simulink model "hyd_hyb_2_opt_cont_test_new_1_col_cd_fin_2"  (Simulink 7.7) (continued)
== system hyd_hyb_2_opt_cont_test_new_1_col_cd_fin_2/controller2/optimized controller/SOC upper 0.5/SOC upper 0.6/SOC upper 0.7/SOC under 0.8/net_loc_rod_7_8/Layer 2/LW{2,1} == (continued)
      mask Display: e = 0.06;c1 = [120 150 230]/255;c2 = ([120 150 230]+0)/383;c3 = ([120 150 230]+128)/383;patch([0 1 1 0],[0 0 1 1],c1);patch([0 1 1 1-e 1-e 0+e],[0 0 1 1-e 0+e 0+e],c2);patch([0 0 1 1-e 0+e 0+e],[0 1 1 1-e 1-e 0+e],c3);plot([0 1 1 0 0],[0 0 1 1 0]);disp('weights');
  "IW{2,1}(39,:)'" : Constant  Value=[-0.4835323749616523;0.31040278631905849;0.10047082836331186;-0.44502359853670193;0.39361966468221593;-0.34187227847267937;-0.18385257985250303;0.063961557191133347;-0.13311223909844291;-0.10037287921349862;-0.022734847026702414;0.32795556493781491;-0.20184661004759019;0.31043902538334567;0.48581448623302109;0.2923177940710513;-0.0082319895963805199;-0.23047279705256349;0.079154749884875836;-0.35425935283586912;-0.33585786795363404;0.55922628182483347;0.024927542637002982;-0.5369677098909581;-0.0095303478724389854;-0.21849709157034933;-0.21380711845089198;0.18488659257561837;0.37658112690975565;-0.098544098121421342]
      mask Display: e = 0.06;c1 = [120 150 230]/255;c2 = ([120 150 230]+0)/383;c3 = ([120 150 230]+128)/383;patch([0 1 1 0],[0 0 1 1],c1);patch([0 1 1 1-e 1-e 0+e],[0 0 1 1-e 0+e 0+e],c2);patch([0 0 1 1-e 0+e 0+e],[0 1 1 1-e 1-e 0+e],c3);plot([0 1 1 0 0],[0 0 1 1 0]);disp('weights');
  "IW{2,1}(4,:)'" : Constant  Value=[-0.084456356670670585;0.39332853492416614;-0.14093791023297814;-0.24699434973438217;0.037218999998135292;0.057290859811489341;-0.078827547784478064;0.49932008441699871;0.16387379971577443;-0.040748085545927626;-0.009324468468041516;-0.19160814023664818;-0.32053131109424393;-0.11908011228852317;-0.041753985083747211;0.26962081886530981;0.46929550230303646;-0.4728704361695264;0.068000938155538287;0.52995252288102757;-0.27876691608214754;0.34925409397203971;-0.023685241942146756;0.1027174484119477;-0.32882096042564896;0.5005590567264776;0.28540974607274233;-0.50723706812721148;0.21128315049192911;0.36676444229034771]
      mask Display: e = 0.06;c1 = [120 150 230]/255;c2 = ([120 150 230]+0)/383;c3 = ([120 150 230]+128)/383;patch([0 1 1 0],[0 0 1 1],c1);patch([0 1 1 1-e 1-e 0+e],[0 0 1 1-e 0+e 0+e],c2);patch([0 0 1 1-e 0+e 0+e],[0 1 1 1-e 1-e 0+e],c3);plot([0 1 1 0 0],[0 0 1 1 0]);disp('weights');
  "IW{2,1}(40,:)'" : Constant  Value=[0.11138375053922149;0.10725746152537521;-0.40441884661729288;-0.37853098423894987;-0.08157669713924344;-0.15157684402681071;0.028628353347344079;-0.43210093459350013;-0.44846964252056087;0.11118148102122939;0.1242481553642565;0.16879590214484513;0.41077554701764851;0.14755675464456003;0.21144023645213661;-0.20514570550553057;-0.44588331488494204;0.40541459720775785;-0.092804745325358151;-0.15342207436425032;0.097208902147965043;0.34979320899396732;0.33451829346632145;-0.16901843840161238;-0.39427988026929328;-0.36030296316647398;0.38700613792454092;-0.31771214900978895;-0.45420022589045494;-0.26445082825380611]
      mask Display: e = 0.06;c1 = [120 150 230]/255;c2 = ([120 150 230]+0)/383;c3 = ([120 150 230]+128)/383;patch([0 1 1 0],[0 0 1 1],c1);patch([0 1 1 1-e 1-e 0+e],[0 0 1 1-e 0+e 0+e],c2);patch([0 0 1 1-e 0+e 0+e],[0 1 1 1-e 1-e 0+e],c3);plot([0 1 1 0 0],[0 0 1 1 0]);disp('weights');
  "IW{2,1}(41,:)'" : Constant  Value=[-0.35630502406955966;-0.36974493824414606;0.15417390376028811;-0.38411362717766467;-0.35707706076629075;0.34165780337971213;0.14516683224012641;-0.21814421311647195;0.0032027169911279991;0.41624788464706797;-0.3397264421764124;-0.34898378374770811;-0.38215900969063754;-0.11404218518317454;-0.0050269406475612136;-0.098560432098857342;0.19486175046204038;0.32148116785508213;-0.38184911577693403;0.18293667936430713;-0.32949787704077893;0.35202275708123654;-0.36473945033324517;0.30077151631589694;0.2861707258441703;-0.080564081375307309;0.42006888197764058;0.11125025887557062;-0.24233566024583689;0.36772368597905775]
      mask Display: e = 0.06;c1 = [120 150 230]/255;c2 = ([120 150 230]+0)/383;c3 = ([120 150 230]+128)/383;patch([0 1 1 0],[0 0 1 1],c1);patch([0 1 1 1-e 1-e 0+e],[0 0 1 1-e 0+e 0+e],c2);patch([0 0 1 1-e 0+e 0+e],[0 1 1 1-e 1-e 0+e],c3);plot([0 1 1 0 0],[0 0 1 1 0]);disp('weights');
  "IW{2,1}(42,:)'" : Constant  Value=[0.15706169119829308;-0.34970464264246925;-0.091467553719374178;0.089096796137939638;0.37726052407804506;-0.45569306047604091;-0.40770262293161275;-0.23251625005436713;-0.25419916471996584;0.061556156981641227;0.38417584029606072;-0.20488679933615037;-0.11108397344155337;0.46208521453680423;-0.16681301652254793;0.009716834658453485;-0.31429738943502228;0.42058563389721992;0.39625395225088711;-0.32712238021736739;0.084481526805292709;-0.29846080219001225;0.07194158791340341;-0.47843218886833982;0.27604781968334097;0.087851162331685273;-0.42601946747014108;-0.17080271868571589;0.209081222714597;-0.25655431363119224]
      mask Display: e = 0.06;c1 = [120 150 230]/255;c2 = ([120 150 230]+0)/383;c3 = ([120 150 230]+128)/383;patch([0 1 1 0],[0 0 1 1],c1);patch([0 1 1 1-e 1-e 0+e],[0 0 1 1-e 0+e 0+e],c2);patch([0 0 1 1-e 0+e 0+e],[0 1 1 1-e 1-e 0+e],c3);plot([0 1 1 0 0],[0 0 1 1 0]);disp('weights');
  "IW{2,1}(43,:)'" : Constant  Value=[-0.31757640025894474;0.056653323308336612;-0.32544780806317231;0.3449113935863426;0.22500001165720365;0.066553259696957981;0.21248106162757274;0.096345459788785906;-0.34025605178291202;-0.44926824011835953;-0.33703884954801289;-0.39135850312812459;0.084811180139392969;0.29004102009119448;-0.066177614890907052;0.2824143243737593;-0.41299189747303039;0.23959623598881055;-0.36516527294692269;-0.029753147329238117;0.03851459755258807;-0.16463600865424638;0.2976532762799749;0.036542029343999097;0.44292641794603299;-0.31092484757236044;-0.14713729631980613;0.41061645318910794;-0.45692360248280139;0.38551952010652746]
      mask Display: e = 0.06;c1 = [120 150 230]/255;c2 = ([120 150 230]+0)/383;c3 = ([120 150 230]+128)/383;patch([0 1 1 0],[0 0 1 1],c1);patch([0 1 1 1-e 1-e 0+e],[0 0 1 1-e 0+e 0+e],c2);patch([0 0 1 1-e 0+e 0+e],[0 1 1 1-e 1-e 0+e],c3);plot([0 1 1 0 0],[0 0 1 1 0]);disp('weights');
  "IW{2,1}(44,:)'" : Constant  Value=[0.27056791623358634;0.08799096571828896;0.40404501597019188;0.22557856196010281;-0.24810705411037701;-0.46898974798625548;-0.48246857128286258;-0.018971563790798295;-0.14180175558241984;-0.11819502415132724;-0.26906558792700436;0.033084771748252563;0.49345840818175823;-0.38563447733884082;-0.24841433722989337;-0.20280384747095245;-0.35483187434085484;0.44982892766393817;0.14130516262448167;0.42433474138815308;-0.067420293627416056;-0.22114163016512606;-0.21059456031308763;-0.1947806306183705;0.082815646444673055;-0.25470852276293143;0.2875592759501811;0.39255425179318121;0.10175831647895807;-0.46482128911740023]
      mask Display: e = 0.06;c1 = [120 150 230]/255;c2 = ([120 150 230]+0)/383;c3 = ([120 150 230]+128)/383;patch([0 1 1 0],[0 0 1 1],c1);patch([0 1 1 1-e 1-e 0+e],[0 0 1 1-e 0+e 0+e],c2);patch([0 0 1 1-e 0+e 0+e],[0 1 1 1-e 1-e 0+e],c3);plot([0 1 1 0 0],[0 0 1 1 0]);disp('weights');
  "IW{2,1}(45,:)'" : Constant  Value=[-0.24278031243361256;0.16460435432975626;0.33695098142409952;-0.38763210309265494;0.11318182663408502;-0.24323292343715391;-0.35412139680927979;-0.48202397097123423;0.34298856029500091;0.45098885155589863;0.29640677932190812;0.12442504925656733;0.22785636922478969;-0.27471140977544156;-0.2568326759436787;-0.33040434674789931;0.40058542584282214;0.28838343142141509;-0.067936896110189549;-0.28159510671003796;0.078580146864540748;0.51250916638014188;0.32989365797735987;-0.26779269746604206;-0.255578957532062;-0.29727334764933683;0.13933585004022109;0.34436541155109002;-0.26846203565570803;-0.32946845076932563]
      mask Display: e = 0.06;c1 = [120 150 230]/255;c2 = ([120 150 230]+0)/383;c3 = ([120 150 230]+128)/383;patch([0 1 1 0],[0 0 1 1],c1);patch([0 1 1 1-e 1-e 0+e],[0 0 1 1-e 0+e 0+e],c2);patch([0 0 1 1-e 0+e 0+e],[0 1 1 1-e 1-e 0+e],c3);plot([0 1 1 0 0],[0 0 1 1 0]);disp('weights');
  "IW{2,1}(46,:)'" : Constant  Value=[0.47751372779563583;-0.004631941129258247;-0.26746797355239715;0.17883186503629808;-0.10548613834212665;0.13687902939422278;-0.40631954704837736;0.17093579645948126;0.095311678944684244;0.034110033251800002;0.3224740096213401;-0.15952180986137474;-0.31784651157245414;-0.45320379549388551;0.33045323818398969;0.22019799015671856;-0.35034858148840908;0.45625883071377721;-0.31719260036143726;-0.50139725511272237;0.1362457531355615;0.22618930416171001;-0.32470450433606052;-0.10633510402694961;-0.31408662865790887;-0.42624423653419202;0.15375441583054122;-0.12959182064022393;0.42217380252437764;-0.25530669324959132]
      mask Display: e = 0.06;c1 = [120 150 230]/255;c2 = ([120 150 230]+0)/383;c3 = ([120 150 230]+128)/383;patch([0 1 1 0],[0 0 1 1],c1);patch([0 1 1 1-e 1-e 0+e],[0 0 1 1-e 0+e 0+e],c2);patch([0 0 1 1-e 0+e 0+e],[0 1 1 1-e 1-e 0+e],c3);plot([0 1 1 0 0],[0 0 1 1 0]);disp('weights');
  "IW{2,1}(47,:)'" : Constant  Value=[-0.33058990973758129;-0.070122866651558854;-0.18964704315156067;0.15987361685157389;0.23026949273690664;0.44625637355610298;0.021772781753390227;-0.053731232580210356;0.08313635696579319;-0.35236494354447639;0.42953438289875867;0.075855817877353904;-0.39252730569594219;0.2648014400238784;0.33156686386675471;0.0039727953447689611;0.34232437544328292;0.42230368814878627;0.38625146824659068;0.37475339723843737;0.39234153591899412;-0.29576359594338791;0.044467362563895552;-0.18506463939778761;0.38881446230224065;-0.34300640961375639;0.31374053810287089;-0.40125736323329159;-0.07249681924861065;-0.26245402643641197]
      mask Display: e = 0.06;c1 = [120 150 230]/255;c2 = ([120 150 230]+0)/383;c3 = ([120 150 230]+128)/383;patch([0 1 1 0],[0 0 1 1],c1);patch([0 1 1 1-e 1-e 0+e],[0 0 1 1-e 0+e 0+e],c2);patch([0 0 1 1-e 0+e 0+e],[0 1 1 1-e 1-e 0+e],c3);plot([0 1 1 0 0],[0 0 1 1 0]);disp('weights');
  "IW{2,1}(48,:)'" : Constant  Value=[-0.0087152117694266774;-0.13863862046810108;0.43608461697351353;0.24508966048687675;-0.31744663574592236;0.037191100287168016;-0.0072845024835617401;0.14337407545120331;0.39230208708277053;-0.26769255922219354;-0.11432636796992721;-0.03563621621721013;-0.45920634652435793;-0.37383833599028848;-0.40166068602002503;-0.039420946924690912;0.47104501996266845;0.2893241869611558;-0.12079466381212473;-0.06437804183397336;-0.20116223091006691;0.19891379096875936;-0.31206785609352838;-0.43312577703778077;0.42758092973608697;-0.33646525622581219;-0.40853609113602746;-0.29060817876817463;-0.25084389770722287;0.34509153925192704]
      mask Display: e = 0.06;c1 = [120 150 230]/255;c2 = ([120 150 230]+0)/383;c3 = ([120 150 230]+128)/383;patch([0 1 1 0],[0 0 1 1],c1);patch([0 1 1 1-e 1-e 0+e],[0 0 1 1-e 0+e 0+e],c2);patch([0 0 1 1-e 0+e 0+e],[0 1 1 1-e 1-e 0+e],c3);plot([0 1 1 0 0],[0 0 1 1 0]);disp('weights');
  "IW{2,1}(49,:)'" : Constant  Value=[0.3664035584933632;0.067770670954671139;-0.50327300088757343;-0.34233762084274327;-0.35402379725705957;-0.19296107393069553;-0.1081773543595337;-0.16307479994707527;-0.4583418301479506;0.22467914290900112;-0.14833729870960458;-0.073885715771716473;-0.018165669962940525;-0.082222221434341144;0.060711633201345906;-0.066032059934777843;0.32062593551943042;-0.39250641268822206;-0.020872485141581968;0.4322512182315113;0.011773603078192489;0.49178856291556711;0.25916934822152971;0.37926759052257847;-0.0021654096910475704;0.15717470271648507;-0.20539255898025252;0.48579751391422438;0.53079080421219049;0.18012053342640411]
      mask Display: e = 0.06;c1 = [120 150 230]/255;c2 = ([120 150 230]+0)/383;c3 = ([120 150 230]+128)/383;patch([0 1 1 0],[0 0 1 1],c1);patch([0 1 1 1-e 1-e 0+e],[0 0 1 1-e 0+e 0+e],c2);patch([0 0 1 1-e 0+e 0+e],[0 1 1 1-e 1-e 0+e],c3);plot([0 1 1 0 0],[0 0 1 1 0]);disp('weights');
  "IW{2,1}(5,:)'" : Constant  Value=[0.42406952511844803;-0.44237375881799146;0.052029662046422891;-0.2036726275790991;-0.16138085114747366;-0.45866443703859072;0.42022368237748842;0.17880090432263859;0.21410679732378329;0.29083240625480378;-0.027317090467041123;0.16565705826923979;0.34867543373333398;0.36985335436086852;0.017207268552833648;0.16536654219096897;0.20655619520552929;0.26425984167836081;-0.22427098686588573;0.44797343941801077;-0.3670662051012662;-0.26225592994412833;0.10611024363293338;0.16269400333774611;0.38885702777445003;0.24951996685976113;-0.401400401142052;-0.34645210174152247;0.41358463544735835;0.0052890876617378317]
      mask Display: e = 0.06;c1 = [120 150 230]/255;c2 = ([120 150 230]+0)/383;c3 = ([120 150 230]+128)/383;patch([0 1 1 0],[0 0 1 1],c1);patch([0 1 1 1-e 1-e 0+e],[0 0 1 1-e 0+e 0+e],c2);patch([0 0 1 1-e 0+e 0+e],[0 1 1 1-e 1-e 0+e],c3);plot([0 1 1 0 0],[0 0 1 1 0]);disp('weights');
  "IW{2,1}(50,:)'" : Constant  Value=[-0.090253487030213214;0.081598635586078078;0.46147121846944167;-0.28886127677024581;0.33883489681456203;0.40165981324062394;-0.56466650847367117;0.40245104763383949;0.20615438894432719;-0.18197549865451798;0.016956460742601938;0.36270102368113011;-0.24568566434979652;0.36504064441923562;0.38856270937240456;-0.2600354429820021;0.14657922287302941;-0.041888408687903327;0.40638754651752573;-0.2321073116173509;-0.39567116183589202;0.33201864852183349;0.25861244614755802;-0.13658164572710443;-0.22688700876353052;0.36437012402211999;-0.36526649346534346;0.067317160225319389;-0.13567612251309988;-0.0042268113432860667]
      mask Display: e = 0.06;c1 = [120 150 230]/255;c2 = ([120 150 230]+0)/383;c3 = ([120 150 230]+128)/383;patch([0 1 1 0],[0 0 1 1],c1);patch([0 1 1 1-e 1-e 0+e],[0 0 1 1-e 0+e 0+e],c2);patch([0 0 1 1-e 0+e 0+e],[0 1 1 1-e 1-e 0+e],c3);plot([0 1 1 0 0],[0 0 1 1 0]);disp('weights');
  "IW{2,1}(51,:)'" : Constant  Value=[-0.41396653348116202;0.34481546560977799;-0.38370551468343417;-0.24768640312877804;-0.18676171803887764;0.084664044981117181;0.18188057084030662;-0.36349132386836369;0.33482426284392841;-0.27228810964946543;0.26642096315480446;-0.30463339503971015;0.43161924106555777;0.42743165422605561;-0.27882990713472916;0.21490073574264024;-0.4056956188624658;-0.32923050103717405;0.090698502245491544;-0.041262366321696824;0.41254424540788398;-0.30370897564739469;0.039541467367836106;0.17200596018618489;-0.26670037961120951;0.27133875503080035;-0.20835111014889679;-0.36281229977734114;-0.39168813383167578;0.052027926329442316]
      mask Display: e = 0.06;c1 = [120 150 230]/255;c2 = ([120 150 230]+0)/383;c3 = ([120 150 230]+128)/383;patch([0 1 1 0],[0 0 1 1],c1);patch([0 1 1 1-e 1-e 0+e],[0 0 1 1-e 0+e 0+e],c2);patch([0 0 1 1-e 0+e 0+e],[0 1 1 1-e 1-e 0+e],c3);plot([0 1 1 0 0],[0 0 1 1 0]);disp('weights');
  "IW{2,1}(52,:)'" : Constant  Value=[-0.38069296020580595;-0.37388677763221584;-0.43527438786809186;-0.10018933070750413;-0.4318270422538768;0.12392134299945326;0.37826031038383068;0.0091844734047052332;0.14314032290612055;-0.09244668315567818;-0.030740872675903589;0.41794729092759891;0.3527559533390181;-0.32905164889004118;-0.13743001235253696;0.3599498134481689;-0.46620731899696716;0.091515812358865661;0.01608888058031591;0.27662234577658446;0.32653038439819637;-0.20897341100774278;-0.3556822785121137;0.19019733497017738;0.18743268464084689;-0.42563891356459305;0.16643055557724315;0.15097951433225962;0.44754909421480576;0.24691090925812617]
      mask Display: e = 0.06;c1 = [120 150 230]/255;c2 = ([120 150 230]+0)/383;c3 = ([120 150 230]+128)/383;patch([0 1 1 0],[0 0 1 1],c1);patch([0 1 1 1-e 1-e 0+e],[0 0 1 1-e 0+e 0+e],c2);patch([0 0 1 1-e 0+e 0+e],[0 1 1 1-e 1-e 0+e],c3);plot([0 1 1 0 0],[0 0 1 1 0]);disp('weights');
  "IW{2,1}(53,:)'" : Constant  Value=[-0.31150996118461921;-0.27089022469089569;0.32394253167930198;-0.191332896808973;-0.047928062465361021;0.2168304813613636;0.41348417125848008;-0.26938956396830288;0.18050331556901592;-0.32919781355229311;0.11232928910461436;0.52365937693549347;0.42875880006211525;0.15022859047575604;0.3944117180161309;-0.41570133018361693;-0.16943344912310337;-0.20083450372814748;0.0097569727379068789;0.44711178267211388;0.14883499873980074;-0.25717842673559238;-0.4027557603667935;-0.50451607457401093;0.061209633399481662;-0.065048057934823808;0.29050523352105062;-0.13416195096729075;-0.058541334279196118;0.40534770083891819]
      mask Display: e = 0.06;c1 = [120 150 230]/255;c2 = ([120 150 230]+0)/383;c3 = ([120 150 230]+128)/383;patch([0 1 1 0],[0 0 1 1],c1);patch([0 1 1 1-e 1-e 0+e],[0 0 1 1-e 0+e 0+e],c2);patch([0 0 1 1-e 0+e 0+e],[0 1 1 1-e 1-e 0+e],c3);plot([0 1 1 0 0],[0 0 1 1 0]);disp('weights');
  "IW{2,1}(54,:)'" : Constant  Value=[-0.29483907497304479;0.2461652272708191;-0.3116494520304734;0.31512836122764426;0.31116596857941942;-0.34272257225836639;0.24982175491241396;-0.13851522175780689;0.4126703301849235;0.45388578747823;-0.19367576526746791;0.38149713324139073;-0.29411255446216084;-0.15238848125567891;-0.10514311756417806;0.38917047266746152;0.34756870536036333;-0.25814615700515281;0.18253749335206168;-0.15105768446019258;0.37290030345230779;-0.10436029482724127;0.088668445563039974;-0.35389946691784768;-0.072190366624104108;0.27381580640706399;0.32273906646128608;-0.11035444148249351;0.44508955593242322;-0.4136644145278352]
      mask Display: e = 0.06;c1 = [120 150 230]/255;c2 = ([120 150 230]+0)/383;c3 = ([120 150 230]+128)/383;patch([0 1 1 0],[0 0 1 1],c1);patch([0 1 1 1-e 1-e 0+e],[0 0 1 1-e 0+e 0+e],c2);patch([0 0 1 1-e 0+e 0+e],[0 1 1 1-e 1-e 0+e],c3);plot([0 1 1 0 0],[0 0 1 1 0]);disp('weights');
  "IW{2,1}(55,:)'" : Constant  Value=[0.39118572971401605;-0.2250950957472681;-0.20171941962608772;0.24447266606081422;0.42651138888792267;0.24439809411326288;-0.43758615906117754;0.037501986208607396;-0.15757758381644171;0.32632471546033442;0.067660957465974572;0.46099293780212147;0.46517367062536269;0.0041267922056686876;-0.49841771824710374;0.2969127225687424;-0.21344262901511921;0.30365400493615929;-0.11538990019070819;-0.092574669250930372;-0.093324047733008411;0.50712233899454184;-0.28998379619409087;0.2334155745609032;0.099475841957016961;0.055594834414815129;-0.0021604909246395665;0.25664450740436828;-0.29261847922183498;0.46213887213115817]
      mask Display: e = 0.06;c1 = [120 150 230]/255;c2 = ([120 150 230]+0)/383;c3 = ([120 150 230]+128)/383;patch([0 1 1 0],[0 0 1 1],c1);patch([0 1 1 1-e 1-e 0+e],[0 0 1 1-e 0+e 0+e],c2);patch([0 0 1 1-e 0+e 0+e],[0 1 1 1-e 1-e 0+e],c3);plot([0 1 1 0 0],[0 0 1 1 0]);disp('weights');
  "IW{2,1}(56,:)'" : Constant  Value=[0.10956897090519425;0.078067756651545819;-0.24671141335522642;-0.33721342820982447;-0.056033239765665509;0.41062236499752036;-0.39699099752884576;0.44969146015822581;0.44650051332625479;0.18436959306935513;0.19283016857168836;-0.28985513531977714;0.2116524262849116;0.10033481493051909;0.42138047710921517;-0.4703061900203232;0.14968360864179775;-0.11481193858597644;0.39424267411879671;0.34748191006008466;0.11095109673002851;-0.061238159900806902;0.40918527279530759;-0.22332485722843232;-0.01006708332520047;0.36222437450209882;0.46192024527265491;0.28260468344650974;0.18372450425154335;-0.17673332315521756]
      mask Display: e = 0.06;c1 = [120 150 230]/255;c2 = ([120 150 230]+0)/383;c3 = ([120 150 230]+128)/383;patch([0 1 1 0],[0 0 1 1],c1);patch([0 1 1 1-e 1-e 0+e],[0 0 1 1-e 0+e 0+e],c2);patch([0 0 1 1-e 0+e 0+e],[0 1 1 1-e 1-e 0+e],c3);plot([0 1 1 0 0],[0 0 1 1 0]);disp('weights');
  "IW{2,1}(57,:)'" : Constant  Value=[0.43804227407036861;-0.5159998225175878;-0.33701718762050747;-0.031017720500566372;-0.14871777161242183;0.10032211889922149;0.29063944480047055;0.25351143938520004;-0.035133259507619766;-0.056715336310332667;-0.40377197613044447;-0.14352543954831098;-0.35572455490944327;-0.44152754392004306;-0.31297546582117852;-0.50986311720027155;-0.24682890604361885;-0.43510966827534103;0.12548325502278765;-0.48710194909433746;-0.036590421752356751;-0.34184634242346773;-0.24358205861594248;-0.30545502383897483;-0.036095338360427061;0.26184896725401141;0.02401899745670423;-0.077217098911994936;0.025681641767577496;0.29518695632614889]
      mask Display: e = 0.06;c1 = [120 150 230]/255;c2 = ([120 150 230]+0)/383;c3 = ([120 150 230]+128)/383;patch([0 1 1 0],[0 0 1 1],c1);patch([0 1 1 1-e 1-e 0+e],[0 0 1 1-e 0+e 0+e],c2);patch([0 0 1 1-e 0+e 0+e],[0 1 1 1-e 1-e 0+e],c3);plot([0 1 1 0 0],[0 0 1 1 0]);disp('weights');
  "IW{2,1}(58,:)'" : Constant  Value=[0.16930085205123677;0.28371315035164307;-0.2470144855589633;0.21249229155357136;-0.0603844950946507;-0.39659481613133929;0.41014133912742501;-0.26557523198499799;-0.43504975054060191;0.39207545976322306;0.24260602150375118;0.42847675400455915;-0.095330919886004395;0.28464677983739034;0.1106155084756012;-0.46936152950406546;-0.28077973095678566;-0.3859824143681162;0.11270371019855152;0.06234339733511287;-0.078391991163682423;0.34866507362632232;0.050136422559474397;0.14960524205469086;0.3595381792035155;-0.44351990189113993;0.15651072103802235;0.22361535349654169;-0.42860118426337152;0.28628469385854016]
      mask Display: e = 0.06;c1 = [120 150 230]/255;c2 = ([120 150 230]+0)/383;c3 = ([120 150 230]+128)/383;patch([0 1 1 0],[0 0 1 1],c1);patch([0 1 1 1-e 1-e 0+e],[0 0 1 1-e 0+e 0+e],c2);patch([0 0 1 1-e 0+e 0+e],[0 1 1 1-e 1-e 0+e],c3);plot([0 1 1 0 0],[0 0 1 1 0]);disp('weights');
  "IW{2,1}(59,:)'" : Constant  Value=[0.013133458356596102;-0.16947000465113868;0.052525881261631262;-0.21148445095941876;-0.027816659817743623;-0.44765098287061333;0.27888652129112274;-0.19898294210082701;-0.44307614058338607;-0.47583075762891147;0.44927750716105758;0.38922229194838248;0.14808235883317414;-0.22702273786493946;0.1488214333108023;0.18174831869052846;0.11783905263004656;0.42033624531995128;-0.17774954126076445;-0.29596628040965173;0.43839517258557292;0.17122752653098278;-0.3024981661667504;0.12473123810865065;0.11513973250108625;-0.35313966621355009;-0.18685425840897918;0.1575305968009536;-0.49353159233018035;0.448366995454752]
      mask Display: e = 0.06;c1 = [120 150 230]/255;c2 = ([120 150 230]+0)/383;c3 = ([120 150 230]+128)/383;patch([0 1 1 0],[0 0 1 1],c1);patch([0 1 1 1-e 1-e 0+e],[0 0 1 1-e 0+e 0+e],c2);patch([0 0 1 1-e 0+e 0+e],[0 1 1 1-e 1-e 0+e],c3);plot([0 1 1 0 0],[0 0 1 1 0]);disp('weights');
  "IW{2,1}(6,:)'" : Constant  Value=[0.21010464111844185;0.12926919188583558;0.56291699377701754;0.14979898609763512;0.47705260514425762;0.20924330541288594;0.053979069364879659;-0.39148496782732456;0.18412309773327401;-0.052234674064068405;-0.077698218736815081;0.2576975729174994;0.079507111213841131;0.42250536873799344;0.28096110125392998;-0.25860390907989156;0.55715333238506881;0.061962856670715619;-0.2088604605645204;-0.32737400587205534;0.11548937129657158;0.28697672119984197;0.61540791806351858;-0.039176868476378648;0.011308823300464415;0.13052017253868084;0.40800611668465114;0.16259908279939292;0.052874027792722086;-0.22259496077489899]
      mask Display: e = 0.06;c1 = [120 150 230]/255;c2 = ([120 150 230]+0)/383;c3 = ([120 150 230]+128)/383;patch([0 1 1 0],[0 0 1 1],c1);patch([0 1 1 1-e 1-e 0+e],[0 0 1 1-e 0+e 0+e],c2);patch([0 0 1 1-e 0+e 0+e],[0 1 1 1-e 1-e 0+e],c3);plot([0 1 1 0 0],[0 0 1 1 0]);disp('weights');
  "IW{2,1}(60,:)'" : Constant  Value=[0.016024547959520175;0.30054613225487642;0.46819975932922692;0.32477253301061015;-0.24984846510593647;0.46239527413244885;-0.28380837242764628;-0.37452279222937757;-0.20947516667758992;0.27883915226055395;-0.12783456571549379;-0.021517592223803561;0.15516629139629934;-0.4411275155019645;0.3672365837654325;0.065536208012601863;0.24329959653357672;-0.38298854091379697;-0.32984530686665808;-0.31336203717149685;-0.051183133090782727;-0.461082886031706;0.28902583018536354;-0.19702489885207919;0.26932184312724489;0.069521434531048878;0.3471931958844186;0.033652027939839457;0.085705869417049496;-0.44890324477768107]
      mask Display: e = 0.06;c1 = [120 150 230]/255;c2 = ([120 150 230]+0)/383;c3 = ([120 150 230]+128)/383;patch([0 1 1 0],[0 0 1 1],c1);patch([0 1 1 1-e 1-e 0+e],[0 0 1 1-e 0+e 0+e],c2);patch([0 0 1 1-e 0+e 0+e],[0 1 1 1-e 1-e 0+e],c3);plot([0 1 1 0 0],[0 0 1 1 0]);disp('weights');
  "IW{2,1}(7,:)'" : Constant  Value=[0.053915427549097193;0.1596965522637947;0.26902295696116257;-0.17339342021059881;0.2606685104231774;0.32570238395898321;-0.055088312681813426;-0.27046632662772219;0.079876293089924791;0.35017269435133452;0.16710614151138262;0.31579823759025782;-0.29834439136182284;-0.18070985569978343;-0.52417852959924827;0.37108115940345238;-0.12263158364146902;-0.4246471823712164;0.47828974260846047;0.39908745108476446;-0.24859678324356294;0.44318829308833074;-0.27387169902745978;-0.2924361399575709;-0.50930123252806347;-0.33273751949952968;-0.036054405229582365;0.12998846643905893;0.035545257274345654;-0.04457124078068532]
      mask Display: e = 0.06;c1 = [120 150 230]/255;c2 = ([120 150 230]+0)/383;c3 = ([120 150 230]+128)/383;patch([0 1 1 0],[0 0 1 1],c1);patch([0 1 1 1-e 1-e 0+e],[0 0 1 1-e 0+e 0+e],c2);patch([0 0 1 1-e 0+e 0+e],[0 1 1 1-e 1-e 0+e],c3);plot([0 1 1 0 0],[0 0 1 1 0]);disp('weights');
  "IW{2,1}(8,:)'" : Constant  Value=[-0.099240137402005629;0.25878094886348363;-0.091868801042810727;-0.30135095888125585;-0.50449824652454511;0.34612873951193368;-0.0010664265809426621;-0.26419275822169624;0.12681942323158812;0.25130761519094069;-0.10613119039260542;-0.1210145857899538;-0.36209531488213426;0.43495748661332079;0.061338249665184169;-0.029357247193802553;0.13779394784072649;0.48322613101242501;0.14386863507301942;-0.09032181714271284;0.49079393686610273;0.12863720993809061;0.34622802166332106;-0.42898847754904523;0.33628940920755962;0.39121943936370257;-0.36796951538815104;-0.038054087692762743;-0.32171101554945147;0.3995732167340193]
      mask Display: e = 0.06;c1 = [120 150 230]/255;c2 = ([120 150 230]+0)/383;c3 = ([120 150 230]+128)/383;patch([0 1 1 0],[0 0 1 1],c1);patch([0 1 1 1-e 1-e 0+e],[0 0 1 1-e 0+e 0+e],c2);patch([0 0 1 1-e 0+e 0+e],[0 1 1 1-e 1-e 0+e],c3);plot([0 1 1 0 0],[0 0 1 1 0]);disp('weights');
  "IW{2,1}(9,:)'" : Constant  Value=[0.37529748219440684;-0.44796060268507837;-0.22584954532295556;-0.095751251308671001;-0.024323043282603866;0.06810181955577202;0.17947727422871981;-0.21143015007561128;0.45550926819454834;0.20387852971357945;-0.40495725927644266;-0.10068818474277683;-0.38347441272242178;-0.33955823499032295;0.24926485440558724;-0.045890003772746574;0.44145216905237583;-0.29324294209613544;-0.021654101945216501;-0.057536183337915776;0.33993952165605285;0.36454274439443385;0.19683473800907428;0.08663986345766006;0.32986231072661071;0.41235912278303605;-0.28620839531444137;0.46658941618340732;-0.029510303842826997;-0.4273621297701255]
      mask Display: e = 0.06;c1 = [120 150 230]/255;c2 = ([120 150 230]+0)/383;c3 = ([120 150 230]+128)/383;patch([0 1 1 0],[0 0 1 1],c1);patch([0 1 1 1-e 1-e 0+e],[0 0 1 1-e 0+e 0+e],c2);patch([0 0 1 1-e 0+e 0+e],[0 1 1 1-e 1-e 0+e],c3);plot([0 1 1 0 0],[0 0 1 1 0]);disp('weights');
  Mux : Mux  Inputs=60
      mask Display: e = 0.06;c1 = [120 150 230]/255;c2 = ([120 150 230]+0)/383;c3 = ([120 150 230]+128)/383;patch([0 1 1 0],[0 0 1 1],c1);patch([0 1 1 1-e 1-e 0+e],[0 0 1 1-e 0+e 0+e],c2);patch([0 0 1 1-e 0+e 0+e],[0 1 1 1-e 1-e 0+e],c3);plot([0 1 1 0 0],[0 0 1 1 0]);disp('Mux');
  dotprod{1..60} (60 alike) : neural/Weight Functions/dotprod  SystemSampleTime=-1
  "lz{2,1}" : Outport
      mask Display: e = 0.06;c1 = [120 230 180]/255;c2 = ([120 230 180]+0)/(256+128);c3 = ([120 230 180]+128)/(256+128);patch([0 1 1 0],[0 0 1 1],c1);patch([0 1 1 1-e 1-e 0+e],[0 0 1 1-e 0+e 0+e],c2);patch([0 0 1 1-e 0+e 0+e],[0 1 1 1-e 1-e 0+e],c3);plot([0 1 1 0 0],[0 0 1 1 0]);
  wires:
  Mux/1 -> "lz{2,1}"/1
  dotprod60/1 -> Mux/60
  "IW{2,1}(60,:)'"/1 -> dotprod60/1
  dotprod59/1 -> Mux/59
  "IW{2,1}(59,:)'"/1 -> dotprod59/1
  dotprod58/1 -> Mux/58
  "IW{2,1}(58,:)'"/1 -> dotprod58/1
  dotprod57/1 -> Mux/57
  "IW{2,1}(57,:)'"/1 -> dotprod57/1
  dotprod56/1 -> Mux/56
  "IW{2,1}(56,:)'"/1 -> dotprod56/1
  dotprod55/1 -> Mux/55
  "IW{2,1}(55,:)'"/1 -> dotprod55/1
  dotprod54/1 -> Mux/54
  "IW{2,1}(54,:)'"/1 -> dotprod54/1
  dotprod53/1 -> Mux/53
  "IW{2,1}(53,:)'"/1 -> dotprod53/1
  dotprod52/1 -> Mux/52
  "IW{2,1}(52,:)'"/1 -> dotprod52/1
  dotprod51/1 -> Mux/51
  "IW{2,1}(51,:)'"/1 -> dotprod51/1
  dotprod50/1 -> Mux/50
  "IW{2,1}(50,:)'"/1 -> dotprod50/1
  dotprod49/1 -> Mux/49
  "IW{2,1}(49,:)'"/1 -> dotprod49/1
  dotprod48/1 -> Mux/48
  "IW{2,1}(48,:)'"/1 -> dotprod48/1
  dotprod47/1 -> Mux/47
  "IW{2,1}(47,:)'"/1 -> dotprod47/1
  dotprod46/1 -> Mux/46
  "IW{2,1}(46,:)'"/1 -> dotprod46/1
  dotprod45/1 -> Mux/45
  "IW{2,1}(45,:)'"/1 -> dotprod45/1
  dotprod44/1 -> Mux/44
  "IW{2,1}(44,:)'"/1 -> dotprod44/1
  dotprod43/1 -> Mux/43
  "IW{2,1}(43,:)'"/1 -> dotprod43/1
  dotprod42/1 -> Mux/42
  "IW{2,1}(42,:)'"/1 -> dotprod42/1
  dotprod41/1 -> Mux/41
  "IW{2,1}(41,:)'"/1 -> dotprod41/1
  dotprod40/1 -> Mux/40
  "IW{2,1}(40,:)'"/1 -> dotprod40/1
  dotprod39/1 -> Mux/39
  "IW{2,1}(39,:)'"/1 -> dotprod39/1
  dotprod38/1 -> Mux/38
  "IW{2,1}(38,:)'"/1 -> dotprod38/1
  dotprod37/1 -> Mux/37
  "IW{2,1}(37,:)'"/1 -> dotprod37/1
  dotprod36/1 -> Mux/36
  "IW{2,1}(36,:)'"/1 -> dotprod36/1
  dotprod35/1 -> Mux/35
  "IW{2,1}(35,:)'"/1 -> dotprod35/1
  dotprod34/1 -> Mux/34
  "IW{2,1}(34,:)'"/1 -> dotprod34/1
  dotprod33/1 -> Mux/33
  "IW{2,1}(33,:)'"/1 -> dotprod33/1
  dotprod32/1 -> Mux/32
  "IW{2,1}(32,:)'"/1 -> dotprod32/1
  dotprod31/1 -> Mux/31
  "IW{2,1}(31,:)'"/1 -> dotprod31/1
  dotprod30/1 -> Mux/30
  "IW{2,1}(30,:)'"/1 -> dotprod30/1
  dotprod29/1 -> Mux/29
  "IW{2,1}(29,:)'"/1 -> dotprod29/1
  dotprod28/1 -> Mux/28
  "IW{2,1}(28,:)'"/1 -> dotprod28/1
  dotprod27/1 -> Mux/27
  "IW{2,1}(27,:)'"/1 -> dotprod27/1
  dotprod26/1 -> Mux/26
  "IW{2,1}(26,:)'"/1 -> dotprod26/1
  dotprod25/1 -> Mux/25
  "IW{2,1}(25,:)'"/1 -> dotprod25/1
  dotprod24/1 -> Mux/24
  "IW{2,1}(24,:)'"/1 -> dotprod24/1
  dotprod23/1 -> Mux/23
  "IW{2,1}(23,:)'"/1 -> dotprod23/1
  dotprod22/1 -> Mux/22
  "IW{2,1}(22,:)'"/1 -> dotprod22/1
  dotprod21/1 -> Mux/21
  "IW{2,1}(21,:)'"/1 -> dotprod21/1
  dotprod20/1 -> Mux/20
  "IW{2,1}(20,:)'"/1 -> dotprod20/1
  dotprod19/1 -> Mux/19
  "IW{2,1}(19,:)'"/1 -> dotprod19/1
  dotprod18/1 -> Mux/18
  "IW{2,1}(18,:)'"/1 -> dotprod18/1
  dotprod17/1 -> Mux/17
  "IW{2,1}(17,:)'"/1 -> dotprod17/1
  dotprod16/1 -> Mux/16
  "IW{2,1}(16,:)'"/1 -> dotprod16/1
  dotprod15/1 -> Mux/15
  "IW{2,1}(15,:)'"/1 -> dotprod15/1
  dotprod14/1 -> Mux/14
  "IW{2,1}(14,:)'"/1 -> dotprod14/1
  dotprod13/1 -> Mux/13
  "IW{2,1}(13,:)'"/1 -> dotprod13/1
  dotprod12/1 -> Mux/12
  "IW{2,1}(12,:)'"/1 -> dotprod12/1
  dotprod11/1 -> Mux/11
  "IW{2,1}(11,:)'"/1 -> dotprod11/1
  dotprod10/1 -> Mux/10
  "IW{2,1}(10,:)'"/1 -> dotprod10/1
  dotprod9/1 -> Mux/9
  "IW{2,1}(9,:)'"/1 -> dotprod9/1
  dotprod8/1 -> Mux/8
  "IW{2,1}(8,:)'"/1 -> dotprod8/1
  dotprod7/1 -> Mux/7
  "IW{2,1}(7,:)'"/1 -> dotprod7/1
  dotprod6/1 -> Mux/6
  "IW{2,1}(6,:)'"/1 -> dotprod6/1
  dotprod5/1 -> Mux/5
  "IW{2,1}(5,:)'"/1 -> dotprod5/1
  dotprod4/1 -> Mux/4
  "IW{2,1}(4,:)'"/1 -> dotprod4/1
  dotprod3/1 -> Mux/3
  "IW{2,1}(3,:)'"/1 -> dotprod3/1
  dotprod2/1 -> Mux/2
  "IW{2,1}(2,:)'"/1 -> dotprod2/1
  dotprod1/1 -> Mux/1
  "ad{2,1}"/1 -> dotprod60/2, dotprod59/2, dotprod58/2, dotprod57/2, dotprod56/2, dotprod55/2, dotprod54/2, dotprod53/2, dotprod52/2, dotprod51/2, dotprod50/2, dotprod49/2, dotprod48/2, dotprod47/2, dotprod46/2, dotprod45/2, dotprod44/2, dotprod43/2, dotprod42/2, dotprod41/2, dotprod40/2, dotprod39/2, dotprod38/2, dotprod37/2, dotprod36/2, dotprod35/2, dotprod34/2, dotprod33/2, dotprod32/2, dotprod31/2, dotprod30/2, dotprod29/2, dotprod28/2, dotprod27/2, dotprod26/2, dotprod25/2, dotprod24/2, dotprod23/2, dotprod22/2, dotprod21/2, dotprod20/2, dotprod19/2, dotprod18/2, dotprod17/2, dotprod16/2, dotprod15/2, dotprod14/2, dotprod13/2, dotprod12/2, dotprod11/2, dotprod10/2, dotprod9/2, dotprod8/2, dotprod7/2, dotprod6/2, dotprod5/2, dotprod4/2, dotprod3/2, dotprod2/2, dotprod1/2
  "IW{2,1}(1,:)'"/1 -> dotprod1/1
